simulink model "AirQlyCtrlr"  (R2022b)
settings: Solver=FixedStepDiscrete  StartTime=0.0  StopTime=10.0  FixedStep=0.2  RelTol=1e-3
settings Model: Creator=blue.ge  LastModifiedBy=blue.ge
settings DataIOCC: SaveFormat=StructureWithTime
settings OptimizationCC: DefaultParameterBehavior=Inlined  EnhancedBackFolding=on  PassReuseOutputArgsAs=Individual arguments  ZeroExternalMemoryAtStartup=off  ZeroInternalMemoryAtStartup=off  OptimizeBlockOrder=speed  DifferentSizesBufferReuse=on  OptimizationCustomize=off  EfficientTunableParamExpr=on
settings DebuggingCC: ParameterTunabilityLossMsg=error
settings ModelReferenceCC: ModelReferenceNumInstancesAllowed=Single
settings SFSimCC: MATLABDynamicMemAlloc=off
settings RTWCC: Description=AUTOSAR  SystemTargetFile=autosar.tlc  GenCodeOnly=on  TemplateMakefile=ert_default_tmf
settings CodeAppCC: ForceParamTrailComments=on  IgnoreCustomStorageClasses=off  OperatorAnnotations=on  SimulinkDataObjDesc=on  SFDataObjDesc=on  InsertBlockDesc=on
settings STFCustomTargetCC: TargetLangStandard=C99 (ISO)  MultiwordLength=256  ParMdlRefBuildCompliant=on  CompOptLevelCompliant=on  IncludeMdlTerminateFcn=off  CombineOutputUpdateFcns=on  SuppressErrorStatus=on  ERTCustomFileBanners=on  SupportNonFinite=off  SupportComplex=off  SupportContinuousTime=off  SupportNonInlinedSFcns=off  RemoveResetFunc=on  MATLABClassNameForMDSCustomization=Simulink.SoftwareTarget.ERTCustomization  AutosarCompliant=on  UseToolchainInfoCompliant=on  LookupTableObjectStructAxisOrder=2,1,3,4,...  LUTObjectStructOrderEvenSpacing=Size,Table,Breakpoints  SystemTargetFile=autosar.tlc
settings ERTTargetCC: TargetLangStandard=C99 (ISO)  ConcurrentExecutionCompliant=off  IncludeMdlTerminateFcn=off  SuppressErrorStatus=on  SupportNonFinite=off  SupportComplex=off  ModelStepFunctionPrototypeControlCompliant=off  CPPClassGenCompliant=off  AutosarCompliant=on  LookupTableObjectStructAxisOrder=2,1,3,4,...  LUTObjectStructOrderEvenSpacing=Size,Table,Breakpoints  InlinedParameterPlacement=NonHierarchical  ERTCustomFileTemplate=example_file_process.tlc

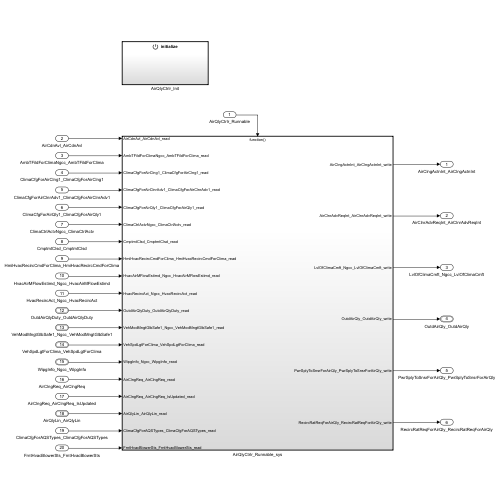
[thumbnail: Simulink's stored preview of the top-level diagram]
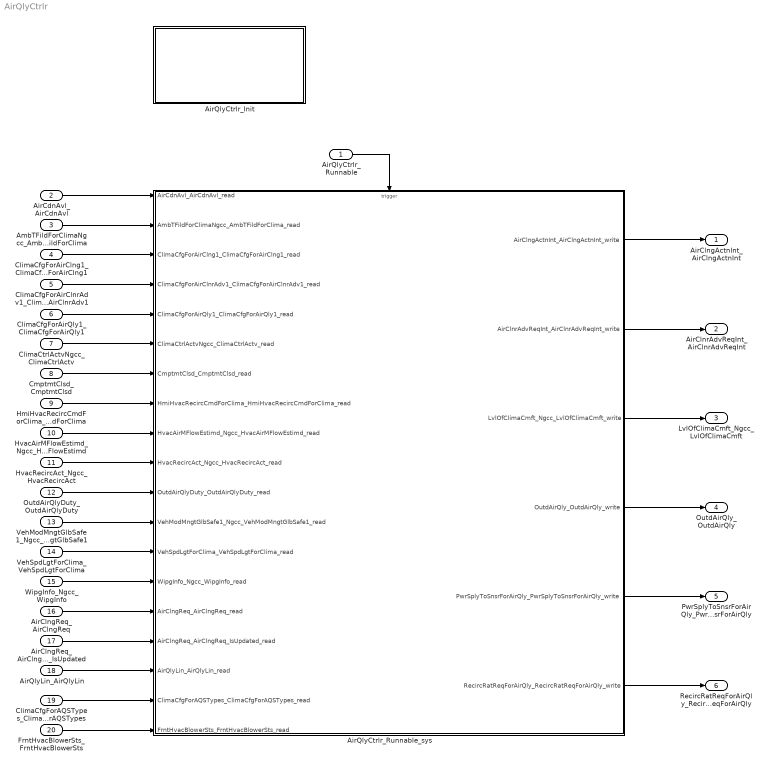
== system AirQlyCtrlr ==
  AirQlyCtrlr_Runnable : Inport  SampleTime=0.2  OutputFunctionCall=on
  AirCdnAvl_AirCdnAvl : Inport  Port=2  OutDataTypeStr=Boolean  PortDimensions=1
  AmbTFildForClimaNgcc_AmbTFildForClima : Inport  Port=3  OutDataTypeStr=T30  PortDimensions=1
  ClimaCfgForAirClng1_ClimaCfgForAirClng1 : Inport  Port=4  OutDataTypeStr=Boolean  PortDimensions=1
  ClimaCfgForAirClnrAdv1_ClimaCfgForAirClnrAdv1 : Inport  Port=5  OutDataTypeStr=Boolean  PortDimensions=1
  ClimaCfgForAirQly1_ClimaCfgForAirQly1 : Inport  Port=6  OutDataTypeStr=Boolean  PortDimensions=1
  ClimaCtrlActvNgcc_ClimaCtrlActv : Inport  Port=7  OutDataTypeStr=Boolean  PortDimensions=1
  CmptmtClsd_CmptmtClsd : Inport  Port=8  OutDataTypeStr=Boolean  PortDimensions=1
  HmiHvacRecircCmdForClima_HmiHvacRecircCmdForClima : Inport  Port=9  OutDataTypeStr=Enum: HmiHvacRecircCmd  PortDimensions=1
  HvacAirMFlowEstimd_Ngcc_HvacAirMFlowEstimd : Inport  Port=10  OutMin=0  OutMax=1000  OutDataTypeStr=MFlow1  PortDimensions=1
  HvacRecircAct_Ngcc_HvacRecircAct : Inport  Port=11  OutDataTypeStr=Perc4  PortDimensions=1
  OutdAirQlyDuty_OutdAirQlyDuty : Inport  Port=12  OutDataTypeStr=Bus: OutdAirQlyDutyWithQly  PortDimensions=1  BusOutputAsStruct=on
  VehModMngtGlbSafe1_Ngcc_VehModMngtGlbSafe1 : Inport  Port=13  OutDataTypeStr=Bus: VehModMngtGlbSafe1  PortDimensions=1  BusOutputAsStruct=on
  VehSpdLgtForClima_VehSpdLgtForClima : Inport  Port=14  OutDataTypeStr=Bus: VehSpdLgtForClima  PortDimensions=1  BusOutputAsStruct=on
  WipgInfo_Ngcc_WipgInfo : Inport  Port=15  OutDataTypeStr=Bus: WipgInfo  PortDimensions=1  BusOutputAsStruct=on
  AirClngReq_AirClngReq : Inport  Port=16  OutDataTypeStr=Enum: OnOffNoReq  PortDimensions=1
  AirClngReq_AirClngReq_IsUpdated : Inport  Port=17  OutDataTypeStr=boolean  PortDimensions=1
  AirQlyLin_AirQlyLin : Inport  Port=18  OutDataTypeStr=Bus: AirQlyLinDutyWithQly  PortDimensions=1  BusOutputAsStruct=on
  ClimaCfgForAQSTypes_ClimaCfgForAQSTypes : Inport  Port=19  OutDataTypeStr=UInt8  PortDimensions=1
  FrntHvacBlowerSts_FrntHvacBlowerSts : Inport  Port=20  OutDataTypeStr=Enum: HvacFanSts  PortDimensions=1
  AirQlyCtrlr_Init : Subsystem
  AirQlyCtrlr_Runnable_sys : Subsystem
  AirClngActnInt_AirClngActnInt : Outport  OutDataTypeStr=Enum: OnOff1  PortDimensions=1
  AirClnrAdvReqInt_AirClnrAdvReqInt : Outport  Port=2  OutDataTypeStr=Enum: AirClnrAdvReqInt  PortDimensions=1
  LvlOfClimaCmft_Ngcc_LvlOfClimaCmft : Outport  Port=3  OutDataTypeStr=Enum: LvlOfClimaCmft  PortDimensions=1
  OutdAirQly_OutdAirQly : Outport  Port=4  OutDataTypeStr=Bus: OutdAirQly  PortDimensions=1  BusOutputAsStruct=on
  PwrSplyToSnsrForAirQly_PwrSplyToSnsrForAirQly : Outport  Port=5  OutDataTypeStr=Enum: OnOff1  PortDimensions=1
  RecircRatReqForAirQly_RecircRatReqForAirQly : Outport  Port=6  OutDataTypeStr=Perc30  PortDimensions=1
  wires:
  AirCdnAvl_AirCdnAvl/1 -> AirQlyCtrlr_Runnable_sys/1(AirCdnAvl_AirCdnAvl_read)
  AmbTFildForClimaNgcc_AmbTFildForClima/1 -> AirQlyCtrlr_Runnable_sys/2(AmbTFildForClimaNgcc_AmbTFildForClima_read)
  ClimaCfgForAirClng1_ClimaCfgForAirClng1/1 -> AirQlyCtrlr_Runnable_sys/3(ClimaCfgForAirClng1_ClimaCfgForAirClng1_read)
  ClimaCfgForAirClnrAdv1_ClimaCfgForAirClnrAdv1/1 -> AirQlyCtrlr_Runnable_sys/4(ClimaCfgForAirClnrAdv1_ClimaCfgForAirClnrAdv1_read)
  ClimaCfgForAirQly1_ClimaCfgForAirQly1/1 -> AirQlyCtrlr_Runnable_sys/5(ClimaCfgForAirQly1_ClimaCfgForAirQly1_read)
  ClimaCtrlActvNgcc_ClimaCtrlActv/1 -> AirQlyCtrlr_Runnable_sys/6(ClimaCtrlActvNgcc_ClimaCtrlActv_read)
  CmptmtClsd_CmptmtClsd/1 -> AirQlyCtrlr_Runnable_sys/7(CmptmtClsd_CmptmtClsd_read)
  HmiHvacRecircCmdForClima_HmiHvacRecircCmdForClima/1 -> AirQlyCtrlr_Runnable_sys/8(HmiHvacRecircCmdForClima_HmiHvacRecircCmdForClima_read)
  HvacAirMFlowEstimd_Ngcc_HvacAirMFlowEstimd/1 -> AirQlyCtrlr_Runnable_sys/9(HvacAirMFlowEstimd_Ngcc_HvacAirMFlowEstimd_read)
  HvacRecircAct_Ngcc_HvacRecircAct/1 -> AirQlyCtrlr_Runnable_sys/10(HvacRecircAct_Ngcc_HvacRecircAct_read)
  OutdAirQlyDuty_OutdAirQlyDuty/1 -> AirQlyCtrlr_Runnable_sys/11(OutdAirQlyDuty_OutdAirQlyDuty_read)
  VehModMngtGlbSafe1_Ngcc_VehModMngtGlbSafe1/1 -> AirQlyCtrlr_Runnable_sys/12(VehModMngtGlbSafe1_Ngcc_VehModMngtGlbSafe1_read)
  VehSpdLgtForClima_VehSpdLgtForClima/1 -> AirQlyCtrlr_Runnable_sys/13(VehSpdLgtForClima_VehSpdLgtForClima_read)
  WipgInfo_Ngcc_WipgInfo/1 -> AirQlyCtrlr_Runnable_sys/14(WipgInfo_Ngcc_WipgInfo_read)
  AirClngReq_AirClngReq/1 -> AirQlyCtrlr_Runnable_sys/15(AirClngReq_AirClngReq_read)
  AirClngReq_AirClngReq_IsUpdated/1 -> AirQlyCtrlr_Runnable_sys/16(AirClngReq_AirClngReq_IsUpdated_read)
  AirQlyLin_AirQlyLin/1 -> AirQlyCtrlr_Runnable_sys/17(AirQlyLin_AirQlyLin_read)
  ClimaCfgForAQSTypes_ClimaCfgForAQSTypes/1 -> AirQlyCtrlr_Runnable_sys/18(ClimaCfgForAQSTypes_ClimaCfgForAQSTypes_read)
  FrntHvacBlowerSts_FrntHvacBlowerSts/1 -> AirQlyCtrlr_Runnable_sys/19(FrntHvacBlowerSts_FrntHvacBlowerSts_read)
  AirQlyCtrlr_Runnable_sys/1(AirClngActnInt_AirClngActnInt_write) -> AirClngActnInt_AirClngActnInt/1
  AirQlyCtrlr_Runnable_sys/2(AirClnrAdvReqInt_AirClnrAdvReqInt_write) -> AirClnrAdvReqInt_AirClnrAdvReqInt/1
  AirQlyCtrlr_Runnable_sys/3(LvlOfClimaCmft_Ngcc_LvlOfClimaCmft_write) -> LvlOfClimaCmft_Ngcc_LvlOfClimaCmft/1
  AirQlyCtrlr_Runnable_sys/4(OutdAirQly_OutdAirQly_write) -> OutdAirQly_OutdAirQly/1
  AirQlyCtrlr_Runnable_sys/5(PwrSplyToSnsrForAirQly_PwrSplyToSnsrForAirQly_write) -> PwrSplyToSnsrForAirQly_PwrSplyToSnsrForAirQly/1
  AirQlyCtrlr_Runnable_sys/6(RecircRatReqForAirQly_RecircRatReqForAirQly_write) -> RecircRatReqForAirQly_RecircRatReqForAirQly/1
  AirQlyCtrlr_Runnable/1 -> AirQlyCtrlr_Runnable_sys/Trigger

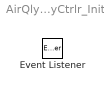
== system AirQlyCtrlr/AirQlyCtrlr_Init ==
  Event Listener : EventListener  EventName=reset

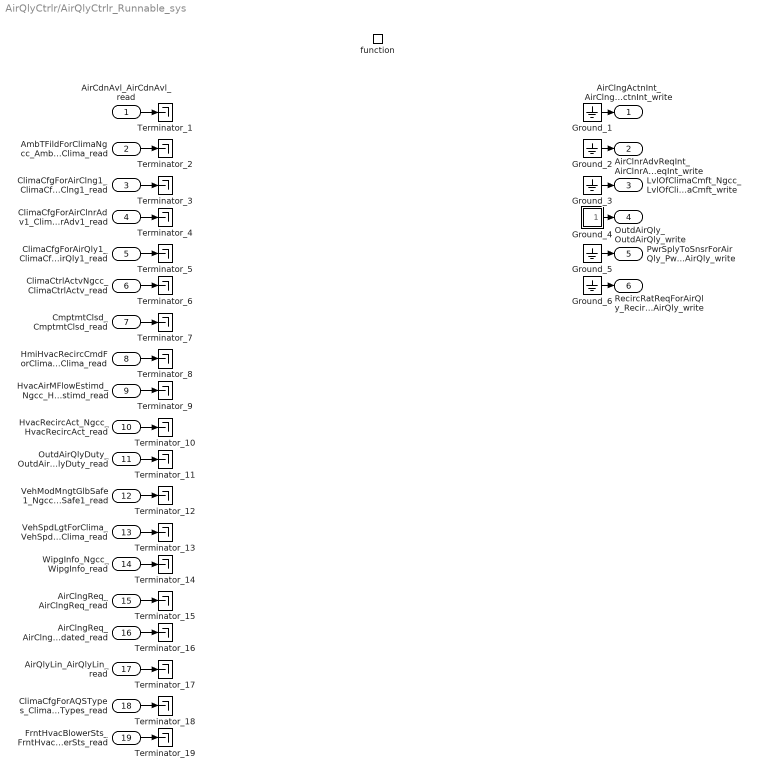
== system AirQlyCtrlr/AirQlyCtrlr_Runnable_sys ==
  AirCdnAvl_AirCdnAvl_read : Inport
  AmbTFildForClimaNgcc_AmbTFildForClima_read : Inport  Port=2
  ClimaCfgForAirClng1_ClimaCfgForAirClng1_read : Inport  Port=3
  ClimaCfgForAirClnrAdv1_ClimaCfgForAirClnrAdv1_read : Inport  Port=4
  ClimaCfgForAirQly1_ClimaCfgForAirQly1_read : Inport  Port=5
  ClimaCtrlActvNgcc_ClimaCtrlActv_read : Inport  Port=6
  CmptmtClsd_CmptmtClsd_read : Inport  Port=7
  HmiHvacRecircCmdForClima_HmiHvacRecircCmdForClima_read : Inport  Port=8
  HvacAirMFlowEstimd_Ngcc_HvacAirMFlowEstimd_read : Inport  Port=9
  HvacRecircAct_Ngcc_HvacRecircAct_read : Inport  Port=10
  OutdAirQlyDuty_OutdAirQlyDuty_read : Inport  Port=11
  VehModMngtGlbSafe1_Ngcc_VehModMngtGlbSafe1_read : Inport  Port=12
  VehSpdLgtForClima_VehSpdLgtForClima_read : Inport  Port=13
  WipgInfo_Ngcc_WipgInfo_read : Inport  Port=14
  AirClngReq_AirClngReq_read : Inport  Port=15
  AirClngReq_AirClngReq_IsUpdated_read : Inport  Port=16
  AirQlyLin_AirQlyLin_read : Inport  Port=17
  ClimaCfgForAQSTypes_ClimaCfgForAQSTypes_read : Inport  Port=18
  FrntHvacBlowerSts_FrntHvacBlowerSts_read : Inport  Port=19
  function : TriggerPort  TriggerType=function-call  VariantControl=(inherit)  SampleTimeType=periodic  SampleTime=-1
  Ground_{1..3,5..6} (5 alike) : Ground
  Ground_4 : Subsystem
  Terminator_{1..19} (19 alike) : Terminator
  AirClngActnInt_AirClngActnInt_write : Outport
      settings: EnsureOutportIsVirtual=on
  AirClnrAdvReqInt_AirClnrAdvReqInt_write : Outport  Port=2
      settings: EnsureOutportIsVirtual=on
  LvlOfClimaCmft_Ngcc_LvlOfClimaCmft_write : Outport  Port=3
      settings: EnsureOutportIsVirtual=on
  OutdAirQly_OutdAirQly_write : Outport  Port=4
      settings: EnsureOutportIsVirtual=on
  PwrSplyToSnsrForAirQly_PwrSplyToSnsrForAirQly_write : Outport  Port=5
      settings: EnsureOutportIsVirtual=on
  RecircRatReqForAirQly_RecircRatReqForAirQly_write : Outport  Port=6
      settings: EnsureOutportIsVirtual=on
  wires:
  AirCdnAvl_AirCdnAvl_read/1 -> Terminator_1/1
  AmbTFildForClimaNgcc_AmbTFildForClima_read/1 -> Terminator_2/1
  ClimaCfgForAirClng1_ClimaCfgForAirClng1_read/1 -> Terminator_3/1
  ClimaCfgForAirClnrAdv1_ClimaCfgForAirClnrAdv1_read/1 -> Terminator_4/1
  ClimaCfgForAirQly1_ClimaCfgForAirQly1_read/1 -> Terminator_5/1
  ClimaCtrlActvNgcc_ClimaCtrlActv_read/1 -> Terminator_6/1
  CmptmtClsd_CmptmtClsd_read/1 -> Terminator_7/1
  HmiHvacRecircCmdForClima_HmiHvacRecircCmdForClima_read/1 -> Terminator_8/1
  HvacAirMFlowEstimd_Ngcc_HvacAirMFlowEstimd_read/1 -> Terminator_9/1
  HvacRecircAct_Ngcc_HvacRecircAct_read/1 -> Terminator_10/1
  OutdAirQlyDuty_OutdAirQlyDuty_read/1 -> Terminator_11/1
  VehModMngtGlbSafe1_Ngcc_VehModMngtGlbSafe1_read/1 -> Terminator_12/1
  VehSpdLgtForClima_VehSpdLgtForClima_read/1 -> Terminator_13/1
  WipgInfo_Ngcc_WipgInfo_read/1 -> Terminator_14/1
  AirClngReq_AirClngReq_read/1 -> Terminator_15/1
  AirClngReq_AirClngReq_IsUpdated_read/1 -> Terminator_16/1
  AirQlyLin_AirQlyLin_read/1 -> Terminator_17/1
  ClimaCfgForAQSTypes_ClimaCfgForAQSTypes_read/1 -> Terminator_18/1
  FrntHvacBlowerSts_FrntHvacBlowerSts_read/1 -> Terminator_19/1
  Ground_1/1 -> AirClngActnInt_AirClngActnInt_write/1
  Ground_2/1 -> AirClnrAdvReqInt_AirClnrAdvReqInt_write/1
  Ground_3/1 -> LvlOfClimaCmft_Ngcc_LvlOfClimaCmft_write/1
  Ground_4/1(Out1) -> OutdAirQly_OutdAirQly_write/1
  Ground_5/1 -> PwrSplyToSnsrForAirQly_PwrSplyToSnsrForAirQly_write/1
  Ground_6/1 -> RecircRatReqForAirQly_RecircRatReqForAirQly_write/1

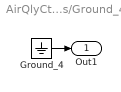
== system AirQlyCtrlr/AirQlyCtrlr_Runnable_sys/Ground_4 ==
  Ground_4 : Ground
  Out1 : Outport  OutDataTypeStr=Bus: OutdAirQly  PortDimensions=1
  wires:
  Ground_4/1 -> Out1/1
